simulink model "PlanarModelandControl"  (R2026a) (continued)
== system PlanarModelandControl/Controle/Gradiente de estabilidade ==
  Cf : Inport
  Vx : Inport  Port=2
  Cr : Inport  Port=3
  1-D Lookup Table : Lookup_n-D  NumberOfTableDimensions=1  BreakpointsForDimension1=Vx  Table=K_variavel  InternalRulePriority=Speed  RndMeth=Simplest
  Divide : Product  Inputs=*/
  Gain : Gain  Gain=lf
  Gain1 : Gain  Gain=lr
  Gain2 : Gain  Gain=2*L
  Gain3 : Gain  Gain=M
  Product : Product
  Subtract : Sum  Inputs=+-
  Subtract1 : Sum  Inputs=+-
  K_ref : Outport
  K_delta : Outport  Port=2
  tag scope: K_atual=global
  wires:
  Cr/1 -> Product/2, Gain1/1
  [K_ref] -> Subtract1/1
  Product/1 -> Gain2/1
  Divide/1 -> [K_atual]
  Subtract1/1 -> K_delta/1
  Gain2/1 -> Divide/2
  [K_atual] -> Subtract1/2
  Gain3/1 -> Divide/1
  Cf/1 -> Gain/1, Product/1
  Gain1/1 -> Subtract/2
  Gain/1 -> Subtract/1
  Subtract/1 -> Gain3/1
  Vx/1 -> 1-D Lookup Table/1
  1-D Lookup Table/1 -> K_ref/1, [K_ref]

== system PlanarModelandControl/Controle/Motores ==
  BusElementIn : Inport  PortName=Omega  Element=fl
  BusElementIn1 : Inport  PortName=Omega  Element=fr
  BusElementIn2 : Inport  PortName=Omega  Element=rl
  BusElementIn3 : Inport  PortName=Omega  Element=rr
  1-D Lookup Table{,1..3} (4 alike) : Lookup_n-D  NumberOfTableDimensions=1  BreakpointsForDimension1=rpm_355_novo  Table=torque_355_novo  InternalRulePriority=Speed  RndMeth=Simplest
  Constant{,1..3} (4 alike) : Constant  Value=Vx_Ref/Re
  Constant{4..7} (4 alike) : Constant  Value=GG_mode
  Gain{,1..3} (4 alike) : Gain  Gain=(60/(2*pi))*Mgt
  Sum : Sum  Inputs=|++++
  Switch{,1..3} (4 alike) : Switch  Criteria=u2 > Threshold  SaturateOnIntegerOverflow=off  InputSameDT=off
  Torque Disponivel : Outport
  wires:
  Gain2/1 -> 1-D Lookup Table2/1
  Constant5/1 -> Switch1/2
  Gain1/1 -> 1-D Lookup Table1/1
  Switch2/1 -> Gain2/1
  Sum/1 -> Torque Disponivel/1
  Gain/1 -> 1-D Lookup Table/1
  Constant1/1 -> Switch1/1
  Switch3/1 -> Gain3/1
  Switch1/1 -> Gain1/1
  1-D Lookup Table1/1 -> Sum/2
  Constant4/1 -> Switch/2
  Constant/1 -> Switch/1
  Gain3/1 -> 1-D Lookup Table3/1
  1-D Lookup Table/1 -> Sum/1
  Constant6/1 -> Switch2/2
  Constant3/1 -> Switch3/1
  1-D Lookup Table3/1 -> Sum/4
  1-D Lookup Table2/1 -> Sum/3
  Switch/1 -> Gain/1
  Constant7/1 -> Switch3/2
  Constant2/1 -> Switch2/1
  BusElementIn/1 -> Switch/3
  BusElementIn1/1 -> Switch1/3
  BusElementIn2/1 -> Switch2/3
  BusElementIn3/1 -> Switch3/3

== system PlanarModelandControl/Controle/Torque Vectoring ==
  note: rever isso daqui
  Pedal map : Inport
  Bus Element In2 : Inport  Port=2  PortName=NormalForces
  Mz : Inport  Port=3
  In Bus Element : Inport  Port=4  PortName=TRC
  Distribuição Lateral2 : Subsystem
  Distribuição longitudinal : Subsystem
  Redução TC1 : Subsystem
  Subsystem : Subsystem
  T_FL : Outport
  T_FR : Outport  Port=2
  T_RL : Outport  Port=3
  T_RR : Outport  Port=4
  wires:
  Redução TC1/3(T_RLreduzido) -> T_RL/1  "RL"
  Subsystem/2("Tq_R ") -> Distribuição Lateral2/4("Tq_R ")
  Mz/1 -> Subsystem/1(MzT)
  Redução TC1/2(T_FRreduzido) -> T_FR/1  "FR"
  Redução TC1/1(T_FLreduzido) -> T_FL/1  "FL"
  Subsystem/1(Tq_F) -> Distribuição Lateral2/3(Tq_F)
  Redução TC1/4(T_RRreduzido) -> T_RR/1  "RR"
  Distribuição longitudinal/1(Dianteiro) -> Distribuição Lateral2/1(Dianteiro)
  Distribuição longitudinal/2(Traseiro) -> Distribuição Lateral2/2(Traseiro)
  Pedal map/1 -> Distribuição longitudinal/2(In1)
  Distribuição Lateral2/1(TFL_lat) -> Redução TC1/2(TFL)
  Distribuição Lateral2/2(TFR_lat) -> Redução TC1/3(TFR)
  Distribuição Lateral2/3(TRL_lat) -> Redução TC1/4(TRL)
  Distribuição Lateral2/4(TRR_lat) -> Redução TC1/5(TRR)
  In Bus Element/1 -> Redução TC1/1(TRC)
  Bus Element In2/1 -> Distribuição longitudinal/1(NormalForces)

== system PlanarModelandControl/Controle/Traction Control ==
  note: Traction Control
  BusElementIn : Inport  PortName=WheelSlips  Element=slip_long_FL
  BusElementIn1 : Inport  PortName=WheelSlips  Element=slip_long_RR
  BusElementIn2 : Inport  PortName=WheelSlips  Element=slip_long_RL
  BusElementIn3 : Inport  PortName=WheelSlips  Element=slip_long_FR
  Rate Transition{1,4..6} (4 alike) : RateTransition  OutPortSampleTime=0.01
  TC_FL : Subsystem
  TC_FR : Subsystem
  TC_RL : Subsystem
  TC_RR : Subsystem
  Zero-Order Hold{1..4} (4 alike) : ZeroOrderHold  SampleTime=0.01
  TRC_FL : Outport
  TRC_FR : Outport  Port=2
  TRC_RL : Outport  Port=3
  TRC_RR : Outport  Port=4
  wires:
  TC_FR/1(Torque reducing coefficient) -> Zero-Order Hold2/1  "Torque reducing coefficient_FR"
  TC_RL/1(Torque reducing coefficient) -> Zero-Order Hold4/1  "Torque reducing coefficient_RL"
  TC_FL/1(Torque reducing coefficient) -> Zero-Order Hold1/1  "Torque reducing coefficient_FL"
  Rate Transition4/1 -> TC_FL/1(wheel slip)
  Rate Transition5/1 -> TC_RL/1(wheel slip)
  Rate Transition6/1 -> TC_RR/1(wheel slip)
  Rate Transition1/1 -> TC_FR/1(wheel slip)
  Zero-Order Hold1/1 -> TRC_FL/1
  Zero-Order Hold4/1 -> TRC_RL/1
  Zero-Order Hold3/1 -> TRC_RR/1
  Zero-Order Hold2/1 -> TRC_FR/1
  TC_RR/1(Torque reducing coefficient) -> Zero-Order Hold3/1  "Torque reducing coefficient_RR"
  BusElementIn/1 -> Rate Transition4/1
  BusElementIn1/1 -> Rate Transition6/1
  BusElementIn2/1 -> Rate Transition5/1
  BusElementIn3/1 -> Rate Transition1/1

== system PlanarModelandControl/Planta/Dinamica do Veículo ==
  Bus Element In1 : Inport  PortName=Driver
  Bus Element In2 : Inport  PortName=Driver
  Bus Element In8 : Inport  Port=2  PortName=CTRL
  In Bus Element{,1..4} (5 alike) : Inport  Port=3  PortName=Movimento
  Dinamica da Roda : Subsystem
  Elipse de tração : Subsystem
  Frenagem : Subsystem
  Load Transfer : Subsystem
  Pacejka : Subsystem
      settings: PortSchema="{\"entries\":[{\"content\":{\"sides\":[{\"content\":{\"connectorIds\":[\"In1\",\"In2\",\"In3\"]},\"type\":\"ConnectorPlacement.EquallySpacedRectSide\",\"uuid\":\"59f4cbcc-dd43-40da-ac6a-8a641ca2d89a\"},{\"content\":{\"connectorIds\":[\"Out1\"],\"side\":\"RIGHT\"},\"type\":\"ConnectorPlacement.EquallySpacedRectSide\",\"uuid\":\"0409e22a-38bf-4c3d-9af5-095aab0f5c69\"},{\"content\":{\"side\":\"TOP\"},\"type\":\"ConnectorPlacement.EquallySpacedRectSide\",\"uuid\":\"002ce310-2645-45d3-8d03-2237c09bbad6\"},{\"content\":{\"connectorIds\":[\"In4\"],\"side\":\"BOTTOM\"},\"type\":\"ConnectorPlacement.EquallySpacedRectSide\",\"uuid\":\"42f47518-e4a8-497c-ae5b-e388981e9179\"}]},\"type\":\"ConnectorPlacement.EquallySpacedConnector\",\"uuid\":\"df842f7d-9145-4d74-853a-61cda4c40c84\"}],\"packageUris\":[\"http://schema.mathworks.com/mf0/Connector/R2020b\"],\"version\":\"1.0\"}"
  Relaxation Length : Subsystem
  Rolling Resistance : Subsystem
  Slip Angle : Subsystem
  Bus Element Out15 : Outport  VectorParamsAs1DForOutWhenUnconnected=off  PortName=Dinamica  Element=Brake
  Out Bus Element : Outport  PortName=Dinamica  Element=NormalForces
  Out Bus Element1 : Outport  PortName=Dinamica  Element=RollingResistances
  Out Bus Element3 : Outport  PortName=Dinamica  Element=LatAndLongForces
  Out Bus Element4 : Outport  PortName=Dinamica  Element=WheelSlips
  Out Bus Element2 : Outport  Port=2  PortName=Omega
  wires:
  Pacejka/1(Pacejka) -> Elipse de tração/1(Pacejka), Dinamica da Roda/4(Pacejka)
  Frenagem/1(Brake) -> Bus Element Out15/1, Dinamica da Roda/1(Brake)
  Dinamica da Roda/1(omega) -> Relaxation Length/3(omega), Out Bus Element2/1
  Bus Element In1/1 -> Frenagem/1(Driver)
  In Bus Element/1 -> Frenagem/2(Movimento)
  In Bus Element1/1 -> Dinamica da Roda/3(Movimento)
  Bus Element In8/1 -> Dinamica da Roda/2(CTRL)
  Bus Element In2/1 -> Slip Angle/1(Driver)
  Dinamica da Roda/2(WheelSlips) -> Out Bus Element4/1, Pacejka/4(WheelSlips)
  In Bus Element2/1 -> Slip Angle/2(Movimento)
  In Bus Element3/1 -> Load Transfer/1(Movimento)
  Load Transfer/1(NormalForces) -> Pacejka/3(NormalForces), Out Bus Element/1, Relaxation Length/2(NormalForces), Rolling Resistance/1(NormalForces)
  In Bus Element4/1 -> Rolling Resistance/2(Movimento)
  Rolling Resistance/1(RollingResistances) -> Out Bus Element1/1
  Slip Angle/1(SlipAnges) -> Pacejka/2(SlipAngles), Relaxation Length/1(SlipAnges)
  Relaxation Length/1(RelaxionLength) -> Pacejka/1(RelaxionLength)
  Elipse de tração/1(LatAndLongForces) -> Out Bus Element3/1

== system PlanarModelandControl/Planta/Movimento do Veiculo ==
  note: Movimento Motor
  Driver : Inport
  Bus Element In1 : Inport  Port=2  PortName=Dinamica
  Bus Element In2 : Inport  Port=2  PortName=Dinamica
  Bus Element In3 : Inport  Port=2  PortName=Dinamica
  In Bus Element : Inport  Port=2  PortName=Dinamica
  In Bus Element1 : Inport  Port=2  PortName=Dinamica
  Bus Creator : BusCreator  Inputs=4
  Gain6 : Gain  Gain=1/R
  Gain7 : Gain  Gain=1/Mgt
  Movimento X : Subsystem
      settings: PortSchema="{\"entries\":[{\"content\":{\"sides\":[{\"content\":{\"connectorIds\":[\"In1\",\"In2\",\"In3\"]},\"type\":\"ConnectorPlacement.EquallySpacedRectSide\",\"uuid\":\"780add47-c131-4723-92e7-967f364f0089\"},{\"content\":{\"connectorIds\":[\"Out1\"],\"side\":\"RIGHT\"},\"type\":\"ConnectorPlacement.EquallySpacedRectSide\",\"uuid\":\"d4af5f03-dec8-406f-b18f-253e126e1949\"},{\"content\":{\"connectorIds\":[\"In4\",\"Out2\"],\"side\":\"TOP\"},\"type\":\"ConnectorPlacement.EquallySpacedRectSide\",\"uuid\":\"57fcaf9d-7236-4cdf-992d-d6b16f62b56e\"},{\"content\":{\"side\":\"BOTTOM\"},\"type\":\"ConnectorPlacement.EquallySpacedRectSide\",\"uuid\":\"5065154b-511f-4bac-af49-ec69d79dc297\"}]},\"type\":\"ConnectorPlacement.EquallySpacedConnector\",\"uuid\":\"d712617a-0f94-443e-927b-9f5aeb9a898b\"}],\"packageUris\":[\"http://schema.mathworks.com/mf0/Connector/R2020b\"],\"version\":\"1.0\"}"
  Movimento Y : Subsystem
      settings: PortSchema="{\"entries\":[{\"content\":{\"sides\":[{\"content\":{\"connectorIds\":[\"In1\",\"In2\",\"In3\"]},\"type\":\"ConnectorPlacement.EquallySpacedRectSide\",\"uuid\":\"780add47-c131-4723-92e7-967f364f0089\"},{\"content\":{\"connectorIds\":[\"Out1\"],\"side\":\"RIGHT\"},\"type\":\"ConnectorPlacement.EquallySpacedRectSide\",\"uuid\":\"d4af5f03-dec8-406f-b18f-253e126e1949\"},{\"content\":{\"side\":\"TOP\"},\"type\":\"ConnectorPlacement.EquallySpacedRectSide\",\"uuid\":\"57fcaf9d-7236-4cdf-992d-d6b16f62b56e\"},{\"content\":{\"connectorIds\":[\"Out2\",\"In4\"],\"side\":\"BOTTOM\"},\"type\":\"ConnectorPlacement.EquallySpacedRectSide\",\"uuid\":\"48ada5c9-a4fe-4454-b4d0-f3a1cb2817a9\"}]},\"type\":\"ConnectorPlacement.EquallySpacedConnector\",\"uuid\":\"d712617a-0f94-443e-927b-9f5aeb9a898b\"}],\"packageUris\":[\"http://schema.mathworks.com/mf0/Connector/R2020b\"],\"version\":\"1.0\"}"
  Ref Inercial - Velocidade : Subsystem
      settings: PortSchema="{\"entries\":[{\"content\":{\"sides\":[{\"content\":{\"connectorIds\":[\"In1\"]},\"type\":\"ConnectorPlacement.EquallySpacedRectSide\",\"uuid\":\"05f8ffe3-bd3b-4bcf-bda5-ceab20fd12dc\"},{\"content\":{\"connectorIds\":[\"In3\"],\"side\":\"RIGHT\"},\"type\":\"ConnectorPlacement.EquallySpacedRectSide\",\"uuid\":\"a57045a4-65cf-4816-a3e6-fda6719b705b\"},{\"content\":{\"side\":\"TOP\"},\"type\":\"ConnectorPlacement.EquallySpacedRectSide\",\"uuid\":\"079956f1-47f0-4de4-8152-820bc1e69142\"},{\"content\":{\"connectorIds\":[\"In2\"],\"side\":\"BOTTOM\"},\"type\":\"ConnectorPlacement.EquallySpacedRectSide\",\"uuid\":\"928d804f-e327-4f58-9490-754ce16cc291\"}]},\"type\":\"ConnectorPlacement.EquallySpacedConnector\",\"uuid\":\"f3948682-b7af-4748-8638-6481b55f2920\"}],\"packageUris\":[\"http://schema.mathworks.com/mf0/Connector/R2020b\"],\"version\":\"1.0\"}"
  Xres : Subsystem
  Yaw : Subsystem
  Yres : Subsystem
  rad/s para rpm4 : Gain  Gain=60/6.283
  Bus Element Out9 : Outport  VectorParamsAs1DForOutWhenUnconnected=off  PortName=Movimento  Element=W_motor
  Bus Element Out4 : Outport  VectorParamsAs1DForOutWhenUnconnected=off  PortName=Movimento  Element=Yaw
  Out Bus Element : Outport  PortName=Movimento  Element=Ay_cg
  Out Bus Element1 : Outport  PortName=Movimento  Element=Ax_cg
  Out Bus Element2 : Outport  PortName=Movimento  Element=Vx_cg
  Out Bus Element3 : Outport  PortName=Movimento  Element=Vy_cg
  wires:
  Gain6/1 -> rad/s para rpm4/1
  rad/s para rpm4/1 -> Gain7/1
  Gain7/1 -> Bus Element Out9/1
  Bus Element In1/1 -> Yaw/1(Dinamica)
  Yaw/1(Yaw) -> Ref Inercial - Velocidade/2(Yaw), Movimento X/1(Yaw), Movimento Y/3(Yaw), Bus Element Out4/1
  Driver/1 -> Yres/1(Driver), Xres/2(Driver)
  Xres/1(Fxref_FL) -> Bus Creator/1  "Fxref_FL"
  Xres/2(Fxref_FR) -> Bus Creator/2  "Fxref_FR"
  Yres/1(Fyref_FL) -> Bus Creator/3  "Fyref_FL"
  Yres/2(Fyref_FR) -> Bus Creator/4  "Fyref_FR"
  Bus Creator/1 -> Movimento Y/1(ProjectedFrontForces), Yaw/2(ProjectedFrontForces), Movimento X/3(ProjectedFrontForces)
  Bus Element In2/1 -> Xres/1(Dinamica)
  Bus Element In3/1 -> Yres/2(Dinamica)
  In Bus Element/1 -> Movimento Y/2(Dinamica)
  In Bus Element1/1 -> Movimento X/2(Dinamica)
  Movimento Y/1(Ay_cg) -> Out Bus Element/1
  Movimento X/1(Ax_cg) -> Out Bus Element1/1
  Movimento X/2(Vx_cg) -> Movimento Y/4(Vx_cg), Gain6/1, Ref Inercial - Velocidade/3(Vx_cg), Out Bus Element2/1  "Vx_cg"
  Movimento Y/2(Vy_cg) -> Out Bus Element3/1, Movimento X/4(Vy_cg), Ref Inercial - Velocidade/1(Vy_cg)  "Vy_cg"

== system PlanarModelandControl/Controle/Direct Yaw Control/MDYC ==
  note: Limite inf e sup do motor
  Yaw_des : Inport
  Vx : Inport  Port=2
  Yaw rate : Inport  Port=3
  Pedal map : Inport  Port=4
  CornR : Inport  Port=5
  CornF : Inport  Port=6
  K delta : Inport  Port=7
  Ay : Inport  Port=8
  Feedback : Subsystem
  Feedfoward : Subsystem
  Limite min : Lookup_n-D  NumberOfTableDimensions=1  BreakpointsForDimension1=pedal  BreakpointsForDimension3=[5, 7]  Table=Mz_minF  InternalRulePriority=Speed  RndMeth=Simplest
  Limite máx : Lookup_n-D  NumberOfTableDimensions=1  BreakpointsForDimension1=pedal  BreakpointsForDimension3=[5, 7]  Table=Mz_maxF  InternalRulePriority=Speed  RndMeth=Simplest
  Saturation Dynamic : sl_discontinuities/Saturation Dynamic  OutMin=[]  OutMax=[]  OutDataTypeStr=Inherit: Same as second input  OutDataType=fixdt(1, 16)  OutScaling=2^-10  DoSatur=off
  Signal Conversion : SignalConversion  OverrideOpt=off
  Sum : Sum  Inputs=|++
  Mz_ajustado : Outport
  wires:
  K delta/1 -> Feedfoward/3(K delta)
  Ay/1 -> Feedfoward/4(Ay)
  CornR/1 -> Feedfoward/1(CornR)
  Yaw rate/1 -> Feedback/1(Yaw rate)
  Vx/1 -> Feedback/2(Vx)
  Yaw_des/1 -> Feedback/3(Yaw_des)
  CornF/1 -> Feedfoward/2(CornF)
  Sum/1 -> Signal Conversion/1
  Limite min/1 -> Saturation Dynamic/3
  Limite máx/1 -> Saturation Dynamic/1
  Pedal map/1 -> Limite min/1, Limite máx/1, Feedback/4(Pedal map), Feedfoward/5(Pedal map)
  Saturation Dynamic/1 -> Mz_ajustado/1
  Signal Conversion/1 -> Saturation Dynamic/2
  Feedback/1(Mz_FB) -> Sum/1
  Feedfoward/1(Mz_FF) -> Sum/2

== system PlanarModelandControl/Controle/Direct Yaw Control/ON//OFF ==
  Volante : Inport
  TV_On : Inport  Port=2
  Mz_ajustado : Inport  Port=3
  Vx : Inport  Port=4
  Abs : Abs  SaturateOnIntegerOverflow=off
  If : If  NumInputs=4  IfExpression=u1<0.05 | u2<1 | u3<2 | u4>0
  If Action Subsystem : Subsystem  TreatAsAtomicUnit=on
  If Action Subsystem1 : Subsystem  TreatAsAtomicUnit=on
  Merge : Merge
  Mz : Outport
  wires:
  Volante/1 -> Abs/1
  Abs/1 -> If/1
  Vx/1 -> If/3
  TV_On/1 -> If/2
  If/1 -> If Action Subsystem/Action
  Mz_ajustado/1 -> If Action Subsystem1/1(Mz_ajustado), If Action Subsystem/1(Mz_ajustado)
  If Action Subsystem/1(Mz_OFF) -> Merge/1
  If Action Subsystem1/1(Mz_ON) -> Merge/2
  Merge/1 -> Mz/1
  [Brake] -> If/4
  If/2 -> If Action Subsystem1/Action

== system PlanarModelandControl/Controle/Direct Yaw Control/Yaw desejado ==
  note: Target Yaw Rate
  note: Yaw Rate Limit
  Steering : Inport
  K ref : Inport  Port=2
  Vx : Inport  Port=3
  Abs : Abs  SaturateOnIntegerOverflow=off
  Abs1 : Abs  SaturateOnIntegerOverflow=off
  Constant : Constant  Value=mi
  Constant1 : Constant  Value=L
  Divide : Product  Inputs=*/
  Divide1 : Product  Inputs=*/
  Gain : Gain  Gain=g
  If : If  NumInputs=2  IfExpression=u2 > u1
  Math Function : Math  Operator=magnitude^2
  Merge : Merge
  Product : Product
  Product1 : Product
  Sum : Sum  Inputs=|++
  ref < limit : Subsystem  TreatAsAtomicUnit=on
  ref > limit : Subsystem  TreatAsAtomicUnit=on
  Yaw_des : Outport
  wires:
  Gain/1 -> Divide/1
  Constant/1 -> Gain/1
  Vx/1 -> Divide1/1, Math Function/1, Abs/1
  Abs/1 -> Divide/2
  Math Function/1 -> Product/1
  K ref/1 -> Product/2
  Product/1 -> Sum/1
  Constant1/1 -> Sum/2
  Sum/1 -> Divide1/2
  Divide1/1 -> Product1/2
  Steering/1 -> Product1/1
  Product1/1 -> ref > limit/2(Yaw_ref), Abs1/1, ref < limit/1(Yaw_ref)
  Divide/1 -> ref > limit/1(Yaw_limit), If/1  "Yaw_limit"
  Abs1/1 -> If/2  "Yaw_ref"
  If/2 -> ref < limit/Action
  If/1 -> ref > limit/Action
  ref < limit/1(Yaw_des) -> Merge/2
  ref > limit/1(Yaw_des) -> Merge/1
  Merge/1 -> Yaw_des/1

== system PlanarModelandControl/Controle/Torque Vectoring/Distribuição Lateral2 ==
  note: Dianteira
  note: Traseira
  Dianteiro : Inport
  Traseiro : Inport  Port=2
  Tq_F : Inport  Port=3
  "Tq_R " : Inport  Port=4
  Saturation{,1..3} (4 alike) : Saturate  UpperLimit=16.2  LowerLimit=0
  Subtract : Sum  Inputs=+-
  Subtract1 : Sum  Inputs=+-
  Sum : Sum  Inputs=|++
  Sum1 : Sum  Inputs=|++
  TFL_lat : Outport
  TFR_lat : Outport  Port=2
  TRL_lat : Outport  Port=3
  TRR_lat : Outport  Port=4
  wires:
  Subtract/1 -> Saturation/1  "TFL"
  "Tq_R "/1 -> Sum1/2, Subtract1/2
  Tq_F/1 -> Sum/2, Subtract/2
  Sum/1 -> Saturation1/1  "TFR"
  Traseiro/1 -> Sum1/1, Subtract1/1
  Sum1/1 -> Saturation3/1  "TRR"
  Subtract1/1 -> Saturation2/1  "TRL"
  Saturation2/1 -> TRL_lat/1  "RL"
  Saturation3/1 -> TRR_lat/1  "RR"
  Dianteiro/1 -> Sum/1, Subtract/1
  Saturation/1 -> TFL_lat/1  "FL"
  Saturation1/1 -> TFR_lat/1  "FR"

== system PlanarModelandControl/Controle/Torque Vectoring/Distribuição longitudinal ==
  BusElementIn : Inport  PortName=NormalForces  Element=Fz_FL
  BusElementIn1 : Inport  PortName=NormalForces  Element=Fz_RL
  BusElementIn2 : Inport  PortName=NormalForces  Element=Fz_RR
  BusElementIn3 : Inport  PortName=NormalForces  Element=Fz_FR
  In1 : Inport  Port=2
  Constant : Constant
  Divide : Product  Inputs=*/
  Gain : Gain  Gain=0.25
  Product : Product
  Product1 : Product
  Sum24 : Sum  Inputs=|++
  Sum25 : Sum  Inputs=|++
  Sum26 : Sum  Inputs=+|+
  Dianteiro : Outport
  Traseiro : Outport  Port=2
  tag scope: Theta=global
  wires:
  Sum24/1 -> Sum26/1, Divide/1
  Divide/1 -> [Theta]
  Sum25/1 -> Sum26/2
  Sum26/1 -> Divide/2
  BusElementIn/1 -> Sum24/1
  BusElementIn2/1 -> Sum25/2
  BusElementIn3/1 -> Sum24/2
  BusElementIn1/1 -> Sum25/1
  Product1/1 -> Traseiro/1
  Product/1 -> Dianteiro/1
  In1/1 -> Gain/1
  Gain/1 -> Product1/1, Product/2
  Constant/1 -> Product/1, Product1/2

== system PlanarModelandControl/Controle/Torque Vectoring/Redução TC1 ==
  BusElementIn : Inport  PortName=TRC  Element=TRC_FL
  BusElementIn1 : Inport  PortName=TRC  Element=TRC_FR
  BusElementIn2 : Inport  PortName=TRC  Element=TRC_RL
  BusElementIn3 : Inport  PortName=TRC  Element=TRC_RR
  TFL : Inport  Port=2
  TFR : Inport  Port=3
  TRL : Inport  Port=4
  TRR : Inport  Port=5
  Constant{1..3,6..7} (5 alike) : Constant
  Product{,1..3} (4 alike) : Product
  Sum{,1..3} (4 alike) : Sum  Inputs=+-|
  Switch{,1..3} (4 alike) : Switch  Criteria=u2 > Threshold  SaturateOnIntegerOverflow=off  InputSameDT=off
  T_FLreduzido : Outport
  T_FRreduzido : Outport  Port=2
  T_RLreduzido : Outport  Port=3
  T_RRreduzido : Outport  Port=4
  wires:
  Constant1/1 -> Sum1/1, Switch/3
  Switch/1 -> Product/2
  Constant3/1 -> Switch1/3, Sum2/1
  Constant7/1 -> Switch2/3, Sum3/1
  Constant6/1 -> Switch3/3
  Switch1/1 -> Product1/2
  TFR/1 -> Product1/1
  Switch2/1 -> Product2/2
  TRL/1 -> Product2/1
  Switch3/1 -> Product3/2
  TRR/1 -> Product3/1
  Product1/1 -> T_FRreduzido/1
  Product/1 -> T_FLreduzido/1
  Product2/1 -> T_RLreduzido/1
  Product3/1 -> T_RRreduzido/1
  TFL/1 -> Product/1
  Sum3/1 -> Switch3/1
  Sum2/1 -> Switch2/1
  Sum/1 -> Switch/1
  Sum1/1 -> Switch1/1
  Constant2/1 -> Sum/1
  BusElementIn/1 -> Switch/2, Sum/2
  BusElementIn1/1 -> Switch1/2, Sum1/2
  BusElementIn2/1 -> Switch2/2, Sum2/2
  BusElementIn3/1 -> Switch3/2, Sum3/2

== system PlanarModelandControl/Controle/Torque Vectoring/Subsystem ==
  MzT : Inport
  Constant : Constant  Value=Re
  Constant1 : Constant  Value=Gr
  Constant2 : Constant  Value=tr
  Divide : Product  Inputs=*/
  Divide1 : Product  Inputs=*/
  Gain : Gain  Gain=2
  Gain1 : Gain  Gain=2
  Gain{2..5} (4 alike) : Gain  Gain=0.5
  Product : Product
  Product1 : Product
  Product2 : Product
  tf : Constant  Value=tf
  Tq_F : Outport
  "Tq_R " : Outport  Port=2
  wires:
  MzT/1 -> Product/2
  Constant/1 -> Product/1
  tf/1 -> Gain4/1
  Constant1/1 -> Product2/1, Product1/2
  Constant2/1 -> Gain5/1
  Product/1 -> Divide/1, Divide1/1
  Product1/1 -> Gain/1
  Product2/1 -> Gain1/1
  Divide/1 -> Gain2/1
  Divide1/1 -> Gain3/1
  Gain/1 -> Divide/2
  Gain1/1 -> Divide1/2
  Gain2/1 -> Tq_F/1
  Gain3/1 -> "Tq_R "/1
  Gain4/1 -> Product1/1
  Gain5/1 -> Product2/2

== system PlanarModelandControl/Controle/Traction Control/TC_FL ==
  wheel slip : Inport
  Fuzzy Controller : Subsystem
  Rate Limiter : RateLimiter  RisingSlewLimit=inf  FallingSlewLimit=-0.5  SampleTimeMode=inherited
  Torque reducing coefficient : Outport
  wires:
  wheel slip/1 -> Fuzzy Controller/1(wheel slip)
  Fuzzy Controller/1(Torque reducing coefficient) -> Rate Limiter/1
  Rate Limiter/1 -> Torque reducing coefficient/1

== system PlanarModelandControl/Controle/Traction Control/TC_FR ==
  wheel slip : Inport
  Fuzzy Controller : Subsystem
  Rate Limiter : RateLimiter  RisingSlewLimit=inf  FallingSlewLimit=-0.5  SampleTimeMode=inherited
  Torque reducing coefficient : Outport
  wires:
  wheel slip/1 -> Fuzzy Controller/1(wheel slip)
  Fuzzy Controller/1(Torque reducing coefficient) -> Rate Limiter/1
  Rate Limiter/1 -> Torque reducing coefficient/1

== system PlanarModelandControl/Controle/Traction Control/TC_RL ==
  wheel slip : Inport
  Fuzzy Controller : Subsystem
  Rate Limiter : RateLimiter  RisingSlewLimit=inf  FallingSlewLimit=-0.5  SampleTimeMode=inherited
  Torque reducing coefficient : Outport
  wires:
  wheel slip/1 -> Fuzzy Controller/1(wheel slip)
  Fuzzy Controller/1(Torque reducing coefficient) -> Rate Limiter/1
  Rate Limiter/1 -> Torque reducing coefficient/1

== system PlanarModelandControl/Controle/Traction Control/TC_RR ==
  wheel slip : Inport
  Fuzzy Controller : Subsystem
  Rate Limiter : RateLimiter  RisingSlewLimit=inf  FallingSlewLimit=-0.5  SampleTimeMode=inherited
  Torque reducing coefficient : Outport
  wires:
  wheel slip/1 -> Fuzzy Controller/1(wheel slip)
  Fuzzy Controller/1(Torque reducing coefficient) -> Rate Limiter/1
  Rate Limiter/1 -> Torque reducing coefficient/1

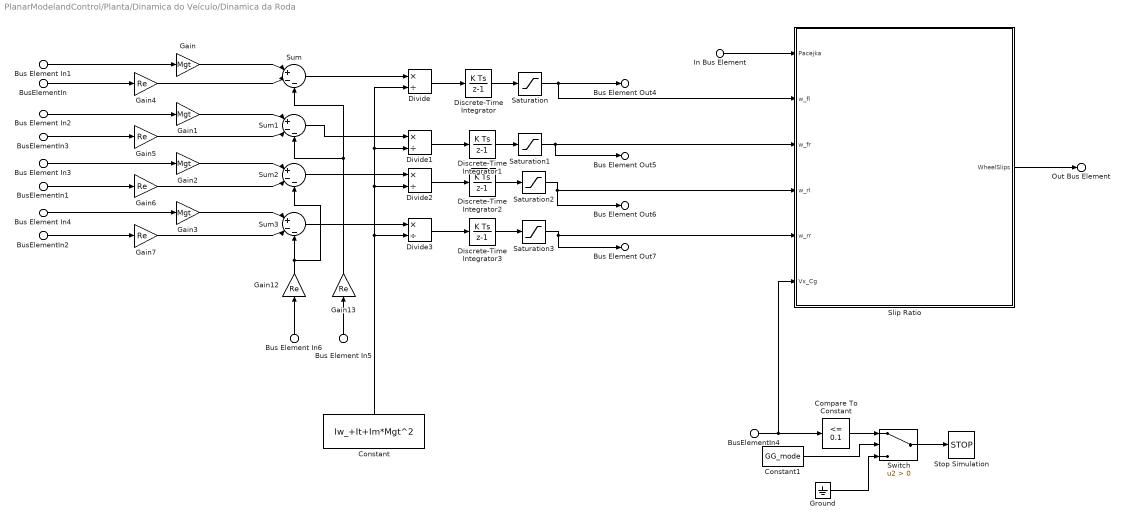
== system PlanarModelandControl/Planta/Dinamica do Veículo/Dinamica da Roda ==
  Bus Element In5 : Inport  PortName=Brake  Element=Fbrake_FL
  Bus Element In6 : Inport  PortName=Brake  Element=Fbrake_RL
  Bus Element In1 : Inport  Port=2  PortName=CTRL  Element=T_FL
  Bus Element In4 : Inport  Port=2  PortName=CTRL  Element=T_RR
  Bus Element In3 : Inport  Port=2  PortName=CTRL  Element=T_RL
  Bus Element In2 : Inport  Port=2  PortName=CTRL  Element=T_FR
  BusElementIn4 : Inport  Port=3  PortName=Movimento  Element=Vx_cg
  In Bus Element : Inport  Port=4  PortName=Pacejka
  BusElementIn : Inport  Port=4  PortName=Pacejka  Element=Fx0_FL
  BusElementIn1 : Inport  Port=4  PortName=Pacejka  Element=Fx0_RL
  BusElementIn2 : Inport  Port=4  PortName=Pacejka  Element=Fx0_RR
  BusElementIn3 : Inport  Port=4  PortName=Pacejka  Element=Fx0_FR
  Compare To Constant : simulink/Logic and Bit Operations/Compare To Constant  relop=<=  const=0.1  OutDataTypeStr=boolean
  Constant : Constant  Value=Iw_+It+Im*Mgt^2
  Constant1 : Constant  Value=GG_mode
  Discrete-Time Integrator{,1..3} (4 alike) : DiscreteIntegrator  InitialCondition=Vx_init/Re  SampleTime=-1  ICPrevOutput=DiscIntNeverNeededParam  ICPrevScaledInput=DiscIntNeverNeededParam
  Divide{,1..3} (4 alike) : Product  Inputs=*/
  Gain{,1..3} (4 alike) : Gain  Gain=Mgt
  Gain{4..7,12..13} (6 alike) : Gain  Gain=Re
  Ground : Ground
  Saturation{,1..3} (4 alike) : Saturate  UpperLimit=inf  LowerLimit=0
  Slip Ratio : Subsystem
  Stop Simulation : Stop
  Sum{,1..3} (4 alike) : Sum  Inputs=|+--
  Switch : Switch  Criteria=u2 > Threshold  SaturateOnIntegerOverflow=off  InputSameDT=off
  Bus Element Out4 : Outport  PortName=omega  Element=fl
  Bus Element Out5 : Outport  PortName=omega  Element=fr
  Bus Element Out6 : Outport  PortName=omega  Element=rl
  Bus Element Out7 : Outport  PortName=omega  Element=rr
  Out Bus Element : Outport  Port=2  PortName=WheelSlips
  wires:
  Gain/1 -> Sum/1
  Gain3/1 -> Sum3/1
  Gain2/1 -> Sum2/1
  Gain1/1 -> Sum1/1
  BusElementIn/1 -> Gain4/1
  BusElementIn3/1 -> Gain5/1
  BusElementIn1/1 -> Gain6/1
  BusElementIn2/1 -> Gain7/1
  Gain4/1 -> Sum/2
  Gain5/1 -> Sum1/2
  Gain6/1 -> Sum2/2
  Gain7/1 -> Sum3/2
  Constant/1 -> Divide3/2, Divide2/2, Divide/2, Divide1/2
  Divide/1 -> Discrete-Time Integrator/1
  Divide1/1 -> Discrete-Time Integrator1/1
  Divide2/1 -> Discrete-Time Integrator2/1
  Divide3/1 -> Discrete-Time Integrator3/1
  Discrete-Time Integrator/1 -> Saturation/1
  Discrete-Time Integrator1/1 -> Saturation1/1
  Discrete-Time Integrator2/1 -> Saturation2/1
  Discrete-Time Integrator3/1 -> Saturation3/1
  Sum/1 -> Divide/1
  Sum1/1 -> Divide1/1
  Sum2/1 -> Divide2/1
  Sum3/1 -> Divide3/1
  Bus Element In5/1 -> Gain13/1
  Gain12/1 -> Sum2/3, Sum3/3
  Gain13/1 -> Sum1/3, Sum/3
  Saturation/1 -> Slip Ratio/2(w_fl), Bus Element Out4/1
  Saturation1/1 -> Bus Element Out5/1, Slip Ratio/3(w_fr)
  Saturation2/1 -> Bus Element Out6/1, Slip Ratio/4(w_rl)
  Saturation3/1 -> Slip Ratio/5(w_rr), Bus Element Out7/1
  BusElementIn4/1 -> Slip Ratio/6(Vx_Cg), Compare To Constant/1
  Compare To Constant/1 -> Switch/1
  Constant1/1 -> Switch/2
  Ground/1 -> Switch/3
  Switch/1 -> Stop Simulation/1
  Bus Element In1/1 -> Gain/1
  Bus Element In2/1 -> Gain1/1
  Bus Element In3/1 -> Gain2/1
  Bus Element In4/1 -> Gain3/1
  Bus Element In6/1 -> Gain12/1
  Slip Ratio/1(WheelSlips) -> Out Bus Element/1
  In Bus Element/1 -> Slip Ratio/1(Pacejka)
